simulink model "r_1f_2x_4a_r340"  (Simulink 7.2) (continued)
== system r_1f_2x_4a_r340 == (continued)
      link override r_2x_8a_r320b_1_instance_id_user_data_out: arith_type=Unsigned  n_bits=32  bin_pt=0  period=1
      link override r_2x_8a_r320b_instance_id_user_data_out: arith_type=Unsigned  n_bits=32  bin_pt=0  period=1
      link override r_4f_1x_16a_r322h_instance_id_user_data_out: arith_type=Unsigned  n_bits=32  bin_pt=0  period=1
      link override r_4f_1x_16a_r322i_instance_id_user_data_out: arith_type=Unsigned  n_bits=32  bin_pt=0  period=1
      link override r_4f_1x_16a_r322j_inst_xeng_id0_user_data_out: arith_type=Unsigned  n_bits=32  bin_pt=0  period=1
      link override r_4f_1x_16a_r322k_inst_xeng_id0_user_data_out: arith_type=Unsigned  n_bits=32  bin_pt=0  period=1
      link override r_4f_1x_16a_r322l_inst_xeng_id0_user_data_out: arith_type=Unsigned  n_bits=32  bin_pt=0  period=1
      link override r_4f_1x_16a_r322m_inst_xeng_id0_user_data_out: arith_type=Unsigned  n_bits=32  bin_pt=0  period=1
      link override r_4f_2x_16a_r320b_instance_id_user_data_out: arith_type=Unsigned  n_bits=32  bin_pt=0  period=1
      link override r_4f_2x_16a_r321a_instance_id_user_data_out: arith_type=Unsigned  n_bits=32  bin_pt=0  period=1
      link override r_4f_2x_16a_r322a_instance_id_user_data_out: arith_type=Unsigned  n_bits=32  bin_pt=0  period=1
      link override r_4f_2x_16a_r322b_instance_id_user_data_out: arith_type=Unsigned  n_bits=32  bin_pt=0  period=1
      link override r_4f_2x_16a_r322c_instance_id_user_data_out: arith_type=Unsigned  n_bits=32  bin_pt=0  period=1
      link override r_4f_2x_16a_r322d_instance_id_user_data_out: arith_type=Unsigned  n_bits=32  bin_pt=0  period=1
      link override r_4f_2x_16a_r322e_instance_id_user_data_out: arith_type=Unsigned  n_bits=32  bin_pt=0  period=1
      link override r_4f_2x_16a_r322f_instance_id_user_data_out: arith_type=Unsigned  n_bits=32  bin_pt=0  period=1
      link override r_4f_2x_16a_r322g_instance_id_user_data_out: arith_type=Unsigned  n_bits=32  bin_pt=0  period=1
      link override r_4f_2x_16a_r322h_instance_id_user_data_out: arith_type=Unsigned  n_bits=32  bin_pt=0  period=1
      link override r_4f_2x_16a_r322m_inst_xeng_id1_user_data_out: arith_type=Unsigned  n_bits=32  bin_pt=0  period=1
      link override r_4f_2x_16a_r323a_inst_xeng_id1_user_data_out: arith_type=Unsigned  n_bits=32  bin_pt=0  period=1
      link override r_4f_2x_16a_r330a_inst_xeng_id1_user_data_out: arith_type=Unsigned  n_bits=32  bin_pt=0  period=1
      link override r_4f_2x_16a_r330b_inst_xeng_id1_user_data_out: arith_type=Unsigned  n_bits=32  bin_pt=0  period=1
      link override r_r311b_xaui_test_instance_id_user_data_out: arith_type=Unsigned  n_bits=32  bin_pt=0  period=1
  last_missing_ant0 : xps_library/software register  Tag=xps:sw_reg  SystemSampleTime=-1  io_dir=To Processor  arith_type=Unsigned  bitwidth=32  bin_pt=0  sample_period=1  gw_name=r_1f_2x_4a_r339_last_missing_ant0_user_data_in
      link override convert: arith_type=Unsigned  n_bits=32  bin_pt=0
  last_missing_ant1 : xps_library/software register  Tag=xps:sw_reg  SystemSampleTime=-1  io_dir=To Processor  arith_type=Unsigned  bitwidth=32  bin_pt=0  sample_period=1  gw_name=r_1f_2x_4a_r339_last_missing_ant1_user_data_in
      link override convert: arith_type=Unsigned  n_bits=32  bin_pt=0
  led0_xaui_fault : xps_library/gpio  Tag=xps:gpio  SystemSampleTime=-1  io_group=ROACH:led  io_dir=out  arith_type=Boolean  bitwidth=1  bin_pt=0  bit_index=0  sample_period=1  use_single_ended=off  use_ddr=off  reg_iob=off  reg_clk_phase=0  termination=None
      link override convert: arith_type=Boolean  n_bits=1  bin_pt=0
  led1_xaui_sync : xps_library/gpio  Tag=xps:gpio  SystemSampleTime=-1  io_group=ROACH:led  io_dir=out  arith_type=Boolean  bitwidth=1  bin_pt=0  bit_index=1  sample_period=1  use_single_ended=off  use_ddr=off  reg_iob=off  reg_clk_phase=0  termination=None
      link override convert: arith_type=Boolean  n_bits=1  bin_pt=0
  led2_x_sync : xps_library/gpio  Tag=xps:gpio  SystemSampleTime=-1  io_group=ROACH:led  io_dir=out  arith_type=Boolean  bitwidth=1  bin_pt=0  bit_index=2  sample_period=1  use_single_ended=off  use_ddr=off  reg_iob=off  reg_clk_phase=0  termination=None
      link override convert: arith_type=Boolean  n_bits=1  bin_pt=0
  led3_new_acc : xps_library/gpio  Tag=xps:gpio  SystemSampleTime=-1  io_group=ROACH:led  io_dir=out  arith_type=Boolean  bitwidth=1  bin_pt=0  bit_index=3  sample_period=1  use_single_ended=off  use_ddr=off  reg_iob=off  reg_clk_phase=0  termination=None
      link override convert: arith_type=Boolean  n_bits=1  bin_pt=0
  loop_cnt0 : xps_library/software register  Tag=xps:sw_reg  SystemSampleTime=-1  io_dir=To Processor  arith_type=Unsigned  bitwidth=32  bin_pt=0  sample_period=1  gw_name=r_1f_2x_4a_r339_loop_cnt0_user_data_in
      link override convert: arith_type=Unsigned  n_bits=32  bin_pt=0
  loop_err_cnt0 : xps_library/software register  Tag=xps:sw_reg  SystemSampleTime=-1  io_dir=To Processor  arith_type=Unsigned  bitwidth=32  bin_pt=0  sample_period=1  gw_name=r_1f_2x_4a_r339_loop_err_cnt0_user_data_in
      link override convert: arith_type=Unsigned  n_bits=32  bin_pt=0
  loopback_fifo0 : Subsystem
  loopback_mux0 : Subsystem  mask{timeout_bits=27, payload_len=32, fifo_latency=4}
      mask prompts: timeout_bits "Timeout (clock cycles, 2^?)"; payload_len "Expected payload length (in 64bit words)"; fifo_latency "Input pkt_fifo latencies"
      mask init:
      | payload_bits = ceil(log2(payload_len)+1);
      | fmtstr = sprintf('Payload Len: %i\nFIFO latencies: %i.', payload_len,fifo_latency);
      | set_param(gcb, 'AttributesFormatString', fmtstr);
      settings: AttributesFormatString=|
      | Payload Len: 32
      | FIFO latencies: 4.
  master_reset_sim2 : Constant  Value=0  OutDataType=sfix(16)  OutScaling=2^0
  master_reset_sim4 : Constant  Value=2  OutDataType=sfix(16)  OutScaling=2^0
  master_reset_sim5 : Constant  Value=0  OutDataType=sfix(16)  OutScaling=2^0
  miss_cnt0 : Subsystem  mask{n_ants_bits=2, xeng_int_bits=7}
      mask prompts: n_ants_bits "Number of antennas in system (2^?)"; xeng_int_bits "X engine integration time (2^?)"
  miss_cnt1 : Subsystem  mask{n_ants_bits=2, xeng_int_bits=7}
      mask prompts: n_ants_bits "Number of antennas in system (2^?)"; xeng_int_bits "X engine integration time (2^?)"
  pkt_reord0 : Subsystem  mask{ant_bits=2, xeng_bits=3, chan_bits=9, x_int_bits=7, window_bits=3, min_sync_bits=27}
      mask prompts: ant_bits "Maximum Number of Antennas (2^?):"; xeng_bits "Number of Xengines in the system (2^?)"; chan_bits "Number of frequency channels in the system (2^?)"; x_int_bits "X Engine Integration Length (2^?):"; window_bits "Number of Windows to Buffer (2^?):"; min_sync_bits "Minimum sync period (2^n)"
      mask init:
      | %sync_bits=max([min_sync_bits,chan_bits+x_int_bits+ant_bits-xeng_bits]);
      | sync_bits=chan_bits+x_int_bits+ant_bits-xeng_bits;
      | fmtstr = sprintf('Sync period: 2^%d\nPayload Len: %i', sync_bits,2^(x_int_bits - 2));
      | set_param(gcb, 'AttributesFormatString', fmtstr);
      settings: AttributesFormatString=|
      | Sync period: 2^15
      | Payload Len: 32
  pkt_reord1 : Subsystem  mask{ant_bits=2, xeng_bits=3, chan_bits=9, x_int_bits=7, window_bits=3, min_sync_bits=27}
      mask prompts: ant_bits "Maximum Number of Antennas (2^?):"; xeng_bits "Number of Xengines in the system (2^?)"; chan_bits "Number of frequency channels in the system (2^?)"; x_int_bits "X Engine Integration Length (2^?):"; window_bits "Number of Windows to Buffer (2^?):"; min_sync_bits "Minimum sync period (2^n)"
      mask init: same as r_1f_2x_4a_r340/pkt_reord0
      settings: AttributesFormatString=|
      | Sync period: 2^15
      | Payload Len: 32
  pkt_reord_cnt0 : xps_library/software register  Tag=xps:sw_reg  SystemSampleTime=-1  io_dir=To Processor  arith_type=Unsigned  bitwidth=32  bin_pt=0  sample_period=1  gw_name=r_1f_2x_4a_r339_pkt_reord_cnt0_user_data_in
      link override convert: arith_type=Unsigned  n_bits=32  bin_pt=0
  pkt_reord_cnt1 : xps_library/software register  Tag=xps:sw_reg  SystemSampleTime=-1  io_dir=To Processor  arith_type=Unsigned  bitwidth=32  bin_pt=0  sample_period=1  gw_name=r_1f_2x_4a_r339_pkt_reord_cnt1_user_data_in
      link override convert: arith_type=Unsigned  n_bits=32  bin_pt=0
  pkt_reord_err0 : xps_library/software register  Tag=xps:sw_reg  SystemSampleTime=-1  io_dir=To Processor  arith_type=Unsigned  bitwidth=32  bin_pt=0  sample_period=1  gw_name=r_1f_2x_4a_r339_pkt_reord_err0_user_data_in
      link override convert: arith_type=Unsigned  n_bits=32  bin_pt=0
  pkt_reord_err1 : xps_library/software register  Tag=xps:sw_reg  SystemSampleTime=-1  io_dir=To Processor  arith_type=Unsigned  bitwidth=32  bin_pt=0  sample_period=1  gw_name=r_1f_2x_4a_r339_pkt_reord_err1_user_data_in
      link override convert: arith_type=Unsigned  n_bits=32  bin_pt=0
  posedge2 : casper_library/Misc/posedge  Tag=Rising Edge Detector  SystemSampleTime=-1
  prep_snap_rx0 : Subsystem  mask{chan_bits=9, xeng_bits=3}
      mask prompts: chan_bits "Number of frequency channels in system (2^?)"; xeng_bits "Number of X engines in the system (2^?)"
  pulse_ext : Subsystem  mask{pulse_len=2^23}
      mask prompts: pulse_len "Length of Pulse"
      mask init:
      | bits = ceil(log2(pulse_len));
  pulse_ext1 : Subsystem  mask{pulse_len=2^23}
      mask prompts: pulse_len "Length of Pulse"
      mask init:
      | bits = ceil(log2(pulse_len));
  pulse_ext2 : Subsystem  mask{pulse_len=2^23}
      mask prompts: pulse_len "Length of Pulse"
      mask init:
      | bits = ceil(log2(pulse_len));
  pulse_ext3 : Subsystem  mask{pulse_len=2^23}
      mask prompts: pulse_len "Length of Pulse"
      mask init:
      | bits = ceil(log2(pulse_len));
  rcs : Subsystem  AncestorBlock=casper_library/Misc/revision_control  mask(revision_control){rev=339, error_on_dirty=off, error_on_norcs=off}
      mask prompts: rev "User revision number"; error_on_dirty "Error on out-of-date"; error_on_norcs "Error on no revision control"
      mask init:
      | revision_control_init( gcb, ...
      |   'rev', rev, ...
      |   'error_on_dirty', error_on_dirty, ...
      |   'error_on_norcs', error_on_norcs);
  rx_cnt0 : xps_library/software register  Tag=xps:sw_reg  SystemSampleTime=-1  io_dir=To Processor  arith_type=Unsigned  bitwidth=32  bin_pt=0  sample_period=1  gw_name=r_1f_2x_4a_r339_rx_cnt0_user_data_in
      link override convert: arith_type=Unsigned  n_bits=32  bin_pt=0
  rx_err_cnt0 : xps_library/software register  Tag=xps:sw_reg  SystemSampleTime=-1  io_dir=To Processor  arith_type=Unsigned  bitwidth=32  bin_pt=0  sample_period=1  gw_name=r_1f_2x_4a_r339_rx_err_cnt0_user_data_in
      link override convert: arith_type=Unsigned  n_bits=32  bin_pt=0
  rx_pkt_fifo0 : Subsystem
      settings: AttributesFormatString=|
      | Max 1024 data words.
      | Max 32 pkts.
  snap_descramble0 : Subsystem  mask(snap_circ){nsamples=11}
      mask prompts: nsamples "Number of Samples (2^?)"
      mask help:
      | Backwards compatible with the normal snap block, but with the following upgrades:
      |  1) Ability to offset the trigger. Optionally delay the fabric trigger by a user-specified number of valids.
      |  2) 'Addr' register's MSb now indicates 'busy capturing'.
      |  3) Ability to capture continuously until a stop pulse is received. Data is captured into BRAM continuously, with old data being overwritten until a stop command is received. Then stop capturing when memory is filled. This enables you to get some data before and some data after the stop pulse. Use the 'tr_en_cnt' register to determine where you are in your vector.
  snap_gbe_rx0 : Subsystem  AncestorBlock=casper_library/Scopes/snap64  mask(snap64){nsamples=11}
      mask prompts: nsamples "No. of samples(2^?)"
      mask help:
      | The <i>snap</i> block provides a packaged solution to capturing data from
      | the FPGA fabric and making it accessible from the CPU.  The block comes in
      | 2 flavors: <i>snap</i> (which captures to a 32 bit wide Shared BRAM), and
      | <i>snap64</i> (which captures to 2x32 bit wide Shared BRAMs to effect a
      | 64 bit capture).
      mask Help: eval('xlWeb([getenv(''MLIB_ROOT''), ''\casper_library\doc\snap_usage.html''])')
  snap_gbe_tx0 : Subsystem  AncestorBlock=casper_library/Scopes/snap64  mask(snap64){nsamples=11}
      mask prompts: nsamples "No. of samples(2^?)"
      mask help:
      | The <i>snap</i> block provides a packaged solution to capturing data from
      | the FPGA fabric and making it accessible from the CPU.  The block comes in
      | 2 flavors: <i>snap</i> (which captures to a 32 bit wide Shared BRAM), and
      | <i>snap64</i> (which captures to 2x32 bit wide Shared BRAMs to effect a
      | 64 bit capture).
      mask Help: eval('xlWeb([getenv(''MLIB_ROOT''), ''\casper_library\doc\snap_usage.html''])')
  snap_rx0 : Subsystem  AncestorBlock=casper_library/Scopes/snap64  mask(snap64){nsamples=12}
      mask prompts: nsamples "No. of samples(2^?)"
      mask help:
      | The <i>snap</i> block provides a packaged solution to capturing data from
      | the FPGA fabric and making it accessible from the CPU.  The block comes in
      | 2 flavors: <i>snap</i> (which captures to a 32 bit wide Shared BRAM), and
      | <i>snap64</i> (which captures to 2x32 bit wide Shared BRAMs to effect a
      | 64 bit capture).
      mask Help: eval('xlWeb([getenv(''MLIB_ROOT''), ''\casper_library\doc\snap_usage.html''])')
  snap_vacc0 : Subsystem  AncestorBlock=casper_library/Scopes/snap  mask(snap){nsamples=11}
      mask prompts: nsamples "No. of samples(2^?)"
      mask help:
      | The <i>snap</i> block provides a packaged solution to capturing data from
      | the FPGA fabric and making it accessible from the CPU.  The block comes in
      | 2 flavors: <i>snap</i> (which captures to a 32 bit wide Shared BRAM), and
      | <i>snap64</i> (which captures to 2x32 bit wide Shared BRAMs to effect a
      | 64 bit capture).
      mask Help: eval('xlWeb([getenv(''MLIB_ROOT''), ''\doc\htmlblocks\sys_snap\sys_snap.html''])')
  snap_vacc1 : Subsystem  AncestorBlock=casper_library/Scopes/snap  mask(snap){nsamples=11}
      mask prompts: nsamples "No. of samples(2^?)"
      mask help:
      | The <i>snap</i> block provides a packaged solution to capturing data from
      | the FPGA fabric and making it accessible from the CPU.  The block comes in
      | 2 flavors: <i>snap</i> (which captures to a 32 bit wide Shared BRAM), and
      | <i>snap64</i> (which captures to 2x32 bit wide Shared BRAMs to effect a
      | 64 bit capture).
      mask Help: eval('xlWeb([getenv(''MLIB_ROOT''), ''\doc\htmlblocks\sys_snap\sys_snap.html''])')
  snap_xaui0 : Subsystem  mask{nsamples=13}
      mask prompts: nsamples "Number of Samples (2^?)"
  snap_xeng0 : Subsystem  mask(snap_circ){nsamples=11}
      mask prompts: nsamples "Number of Samples (2^?)"
      mask help:
      | Backwards compatible with the normal snap block, but with the following upgrades:
      |  1) Ability to offset the trigger. Optionally delay the fabric trigger by a user-specified number of valids.
      |  2) 'Addr' register's MSb now indicates 'busy capturing'.
      |  3) Ability to capture continuously until a stop pulse is received. Data is captured into BRAM continuously, with old data being overwritten until a stop command is received. Then stop capturing when memory is filled. This enables you to get some data before and some data after the stop pulse. Use the 'tr_en_cnt' register to determine where you are in your vector.
  tvg_sel : xps_library/software register  Tag=xps:sw_reg  SystemSampleTime=-1  io_dir=From Processor  arith_type=Unsigned  bitwidth=32  bin_pt=0  sample_period=1  gw_name=r_1f_2x_4a_r339_tvg_sel_user_data_out
      link override r_2x_8a_r310b_tvg_sel1_user_data_out: arith_type=Unsigned  n_bits=32  bin_pt=0  period=1
      link override r_2x_8a_r311a_tvg_sel1_user_data_out: arith_type=Unsigned  n_bits=32  bin_pt=0  period=1
      link override r_2x_8a_r311b_1_tvg_sel_user_data_out: arith_type=Unsigned  n_bits=32  bin_pt=0  period=1
      link override r_2x_8a_r311b_tvg_sel_user_data_out: arith_type=Unsigned  n_bits=32  bin_pt=0  period=1
      link override r_2x_8a_r320a_1_tvg_sel_user_data_out: arith_type=Unsigned  n_bits=32  bin_pt=0  period=1
      link override r_2x_8a_r320b_1_tvg_sel_user_data_out: arith_type=Unsigned  n_bits=32  bin_pt=0  period=1
      link override r_2x_8a_r320b_tvg_sel_user_data_out: arith_type=Unsigned  n_bits=32  bin_pt=0  period=1
      link override r_4f_1x_16a_r322h_tvg_sel_user_data_out: arith_type=Unsigned  n_bits=32  bin_pt=0  period=1
      link override r_4f_1x_16a_r322i_tvg_sel_user_data_out: arith_type=Unsigned  n_bits=32  bin_pt=0  period=1
      link override r_4f_1x_16a_r322j_tvg_sel_user_data_out: arith_type=Unsigned  n_bits=32  bin_pt=0  period=1
      link override r_4f_1x_16a_r322k_tvg_sel_user_data_out: arith_type=Unsigned  n_bits=32  bin_pt=0  period=1
      link override r_4f_1x_16a_r322l_tvg_sel_user_data_out: arith_type=Unsigned  n_bits=32  bin_pt=0  period=1
      link override r_4f_1x_16a_r322m_tvg_sel_user_data_out: arith_type=Unsigned  n_bits=32  bin_pt=0  period=1
      link override r_4f_2x_16a_r320b_tvg_sel_user_data_out: arith_type=Unsigned  n_bits=32  bin_pt=0  period=1
      link override r_4f_2x_16a_r321a_tvg_sel_user_data_out: arith_type=Unsigned  n_bits=32  bin_pt=0  period=1
      link override r_4f_2x_16a_r322a_tvg_sel_user_data_out: arith_type=Unsigned  n_bits=32  bin_pt=0  period=1
      link override r_4f_2x_16a_r322b_tvg_sel_user_data_out: arith_type=Unsigned  n_bits=32  bin_pt=0  period=1
      link override r_4f_2x_16a_r322c_tvg_sel_user_data_out: arith_type=Unsigned  n_bits=32  bin_pt=0  period=1
      link override r_4f_2x_16a_r322d_tvg_sel_user_data_out: arith_type=Unsigned  n_bits=32  bin_pt=0  period=1
      link override r_4f_2x_16a_r322e_tvg_sel_user_data_out: arith_type=Unsigned  n_bits=32  bin_pt=0  period=1
      link override r_4f_2x_16a_r322f_tvg_sel_user_data_out: arith_type=Unsigned  n_bits=32  bin_pt=0  period=1
      link override r_4f_2x_16a_r322g_tvg_sel_user_data_out: arith_type=Unsigned  n_bits=32  bin_pt=0  period=1
      link override r_4f_2x_16a_r322h_tvg_sel_user_data_out: arith_type=Unsigned  n_bits=32  bin_pt=0  period=1
      link override r_4f_2x_16a_r322m_tvg_sel_user_data_out: arith_type=Unsigned  n_bits=32  bin_pt=0  period=1
      link override r_4f_2x_16a_r323a_tvg_sel_user_data_out: arith_type=Unsigned  n_bits=32  bin_pt=0  period=1
      link override r_4f_2x_16a_r330a_tvg_sel_user_data_out: arith_type=Unsigned  n_bits=32  bin_pt=0  period=1
      link override r_4f_2x_16a_r330b_tvg_sel_user_data_out: arith_type=Unsigned  n_bits=32  bin_pt=0  period=1
      link override r_r311b_xaui_test_tvg_sel_user_data_out: arith_type=Unsigned  n_bits=32  bin_pt=0  period=1
      link override test_x_eng_nw_sim_pkt_4a_10_1_tvg_sel_user_data_out: arith_type=Unsigned  n_bits=32  bin_pt=0  period=1
      link override test_x_eng_sim_packet_4a_10_1_tvg_sel_user_data_out: arith_type=Unsigned  n_bits=32  bin_pt=0  period=1
      link override test_x_eng_sim_pkt_4a_10_1_new_tvg_sel_user_data_out: arith_type=Unsigned  n_bits=32  bin_pt=0  period=1
      link override xflow_sim_8a_pt2_tvg_sel_user_data_out: arith_type=Unsigned  n_bits=32  bin_pt=0  period=1
      link override xflow_sim_8a_tvg_sel_user_data_out: arith_type=Unsigned  n_bits=32  bin_pt=0  period=1
      link override xflow_sim_tvg_sel_user_data_out: arith_type=Unsigned  n_bits=32  bin_pt=0  period=1
  vacc0 : Subsystem  mask{ants_bits=2, freq_bits=9, xeng_bits=3, which_qdr=qdr0}
      mask prompts: ants_bits "Number of antennas (2^n)"; freq_bits "Number of frequency channels (2^n)"; xeng_bits "Number of X engines (2^n)"; which_qdr "QDR"
      mask help: Hardcoded for X engines with a demux of 8!
      mask init:
      | vector_len = (2^ants_bits)*(2^ants_bits +1) /2 *8 * 2^(freq_bits-xeng_bits)
      | set_param([gcb, '/qv/qdr'], 'which_qdr', which_qdr);
      | fmtstr = sprintf('Vector Length: %d', vector_len);
      | set_param(gcb, 'AttributesFormatString', fmtstr);
      settings: AttributesFormatString=Vector Length: 5120
      mask StyleString: edit,edit,edit,popup(qdr0|qdr1)
  vacc1 : Subsystem  mask{ants_bits=2, freq_bits=9, xeng_bits=3, which_qdr=qdr1}
      mask prompts: ants_bits "Number of antennas (2^n)"; freq_bits "Number of frequency channels (2^n)"; xeng_bits "Number of X engines (2^n)"; which_qdr "QDR"
      mask help: Hardcoded for X engines with a demux of 8!
      mask init: same as r_1f_2x_4a_r340/vacc0
      settings: AttributesFormatString=Vector Length: 5120
      mask StyleString: edit,edit,edit,popup(qdr0|qdr1)
  vacc_cnt0 : xps_library/software register  Tag=xps:sw_reg  SystemSampleTime=-1  io_dir=To Processor  arith_type=Unsigned  bitwidth=32  bin_pt=0  sample_period=1  gw_name=r_1f_2x_4a_r339_vacc_cnt0_user_data_in
      link override convert: arith_type=Unsigned  n_bits=32  bin_pt=0
  vacc_cnt1 : xps_library/software register  Tag=xps:sw_reg  SystemSampleTime=-1  io_dir=To Processor  arith_type=Unsigned  bitwidth=32  bin_pt=0  sample_period=1  gw_name=r_1f_2x_4a_r339_vacc_cnt1_user_data_in
      link override convert: arith_type=Unsigned  n_bits=32  bin_pt=0
  vacc_err_cnt0 : xps_library/software register  Tag=xps:sw_reg  SystemSampleTime=-1  io_dir=To Processor  arith_type=Unsigned  bitwidth=32  bin_pt=0  sample_period=1  gw_name=r_1f_2x_4a_r339_vacc_err_cnt0_user_data_in
      link override convert: arith_type=Unsigned  n_bits=32  bin_pt=0
  vacc_err_cnt1 : xps_library/software register  Tag=xps:sw_reg  SystemSampleTime=-1  io_dir=To Processor  arith_type=Unsigned  bitwidth=32  bin_pt=0  sample_period=1  gw_name=r_1f_2x_4a_r339_vacc_err_cnt1_user_data_in
      link override convert: arith_type=Unsigned  n_bits=32  bin_pt=0
  vacc_ld_status0 : xps_library/software register  Tag=xps:sw_reg  SystemSampleTime=-1  io_dir=To Processor  arith_type=Unsigned  bitwidth=32  bin_pt=0  sample_period=1  gw_name=r_1f_2x_4a_r339_vacc_ld_status0_user_data_in
      link override convert: arith_type=Unsigned  n_bits=32  bin_pt=0
  vacc_ld_status1 : xps_library/software register  Tag=xps:sw_reg  SystemSampleTime=-1  io_dir=To Processor  arith_type=Unsigned  bitwidth=32  bin_pt=0  sample_period=1  gw_name=r_1f_2x_4a_r339_vacc_ld_status1_user_data_in
      link override convert: arith_type=Unsigned  n_bits=32  bin_pt=0
  vacc_mcnt_h0 : xps_library/software register  Tag=xps:sw_reg  SystemSampleTime=-1  io_dir=To Processor  arith_type=Unsigned  bitwidth=32  bin_pt=0  sample_period=1  gw_name=r_1f_2x_4a_r339_vacc_mcnt_h0_user_data_in
      link override convert: arith_type=Unsigned  n_bits=32  bin_pt=0
  vacc_mcnt_h1 : xps_library/software register  Tag=xps:sw_reg  SystemSampleTime=-1  io_dir=To Processor  arith_type=Unsigned  bitwidth=32  bin_pt=0  sample_period=1  gw_name=r_1f_2x_4a_r339_vacc_mcnt_h1_user_data_in
      link override convert: arith_type=Unsigned  n_bits=32  bin_pt=0
  vacc_mcnt_l0 : xps_library/software register  Tag=xps:sw_reg  SystemSampleTime=-1  io_dir=To Processor  arith_type=Unsigned  bitwidth=32  bin_pt=0  sample_period=1  gw_name=r_1f_2x_4a_r339_vacc_mcnt_l0_user_data_in
      link override convert: arith_type=Unsigned  n_bits=32  bin_pt=0
  vacc_mcnt_l1 : xps_library/software register  Tag=xps:sw_reg  SystemSampleTime=-1  io_dir=To Processor  arith_type=Unsigned  bitwidth=32  bin_pt=0  sample_period=1  gw_name=r_1f_2x_4a_r339_vacc_mcnt_l1_user_data_in
      link override convert: arith_type=Unsigned  n_bits=32  bin_pt=0
  vacc_monitor : Subsystem
  vacc_monitor1 : Subsystem
  vacc_time_lsw : xps_library/software register  Tag=xps:sw_reg  SystemSampleTime=-1  io_dir=From Processor  arith_type=Unsigned  bitwidth=32  bin_pt=0  sample_period=1  gw_name=r_1f_2x_4a_r339_vacc_time_lsw_user_data_out
      link override poco_cd_tr0_ld_time_lsb_user_data_out: arith_type=Unsigned  n_bits=32  bin_pt=0  period=1
      link override poco_dram_vacc_r001_cd_tr0_ld_time_lsb_user_data_out: arith_type=Unsigned  n_bits=32  bin_pt=0  period=1
      link override poco_dram_vacc_r002_cd_tr0_ld_time_lsb_user_data_out: arith_type=Unsigned  n_bits=32  bin_pt=0  period=1
      link override poco_dram_vacc_r003_cd_tr0_ld_time_lsb_user_data_out: arith_type=Unsigned  n_bits=32  bin_pt=0  period=1
      link override poco_dram_vacc_r004_cd_tr0_ld_time_lsb_user_data_out: arith_type=Unsigned  n_bits=32  bin_pt=0  period=1
      link override poco_dram_vacc_r005_cd_tr0_ld_time_lsb_user_data_out: arith_type=Unsigned  n_bits=32  bin_pt=0  period=1
      link override poco_dram_vacc_r006_cd_tr0_ld_time_lsb_user_data_out: arith_type=Unsigned  n_bits=32  bin_pt=0  period=1
      link override poco_r006_cd_tr0_ld_time_lsb_user_data_out: arith_type=Unsigned  n_bits=32  bin_pt=0  period=1
      link override poco_red_cd_ld_time_lsb_user_data_out: arith_type=Unsigned  n_bits=32  bin_pt=0  period=1
      link override poco_ref_roach_adc0_cd_ld_time_lsb_user_data_out: arith_type=Unsigned  n_bits=32  bin_pt=0  period=1
      link override poco_ref_roach_reduced_adc0_cd_ld_time_lsb_user_data_out: arith_type=Unsigned  n_bits=32  bin_pt=0  period=1
      link override poco_roach_cd_tr0_ld_time_lsb_user_data_out: arith_type=Unsigned  n_bits=32  bin_pt=0  period=1
      link override poco_roach_r006_cd_tr0_ld_time_lsb_user_data_out: arith_type=Unsigned  n_bits=32  bin_pt=0  period=1
      link override poco_roach_r007_cd_tr0_ld_time_lsb_user_data_out: arith_type=Unsigned  n_bits=32  bin_pt=0  period=1
      link override poco_roach_red_adc0_cd_ld_time_lsb_user_data_out: arith_type=Unsigned  n_bits=32  bin_pt=0  period=1
      link override poco_roach_vacc_r006_cd_tr0_ld_time_lsb_user_data_out: arith_type=Unsigned  n_bits=32  bin_pt=0  period=1
  vacc_time_msw : xps_library/software register  Tag=xps:sw_reg  SystemSampleTime=-1  io_dir=From Processor  arith_type=Unsigned  bitwidth=32  bin_pt=0  sample_period=1  gw_name=r_1f_2x_4a_r339_vacc_time_msw_user_data_out
      link override poco_cd_tr0_ld_time_msb_user_data_out: arith_type=Unsigned  n_bits=32  bin_pt=0  period=1
      link override poco_dram_vacc_r001_cd_tr0_ld_time_msb_user_data_out: arith_type=Unsigned  n_bits=32  bin_pt=0  period=1
      link override poco_dram_vacc_r002_cd_tr0_ld_time_msb_user_data_out: arith_type=Unsigned  n_bits=32  bin_pt=0  period=1
      link override poco_dram_vacc_r003_cd_tr0_ld_time_msb_user_data_out: arith_type=Unsigned  n_bits=32  bin_pt=0  period=1
      link override poco_dram_vacc_r004_cd_tr0_ld_time_msb_user_data_out: arith_type=Unsigned  n_bits=32  bin_pt=0  period=1
      link override poco_dram_vacc_r005_cd_tr0_ld_time_msb_user_data_out: arith_type=Unsigned  n_bits=32  bin_pt=0  period=1
      link override poco_dram_vacc_r006_cd_tr0_ld_time_msb_user_data_out: arith_type=Unsigned  n_bits=32  bin_pt=0  period=1
      link override poco_r006_cd_tr0_ld_time_msb_user_data_out: arith_type=Unsigned  n_bits=32  bin_pt=0  period=1
      link override poco_red_cd_ld_time_msb_user_data_out: arith_type=Unsigned  n_bits=32  bin_pt=0  period=1
      link override poco_ref_roach_adc0_cd_ld_time_msb_user_data_out: arith_type=Unsigned  n_bits=32  bin_pt=0  period=1
      link override poco_ref_roach_reduced_adc0_cd_ld_time_msb_user_data_out: arith_type=Unsigned  n_bits=32  bin_pt=0  period=1
      link override poco_roach_cd_tr0_ld_time_msb_user_data_out: arith_type=Unsigned  n_bits=32  bin_pt=0  period=1
      link override poco_roach_r006_cd_tr0_ld_time_msb_user_data_out: arith_type=Unsigned  n_bits=32  bin_pt=0  period=1
      link override poco_roach_r007_cd_tr0_ld_time_msb_user_data_out: arith_type=Unsigned  n_bits=32  bin_pt=0  period=1
      link override poco_roach_red_adc0_cd_ld_time_msb_user_data_out: arith_type=Unsigned  n_bits=32  bin_pt=0  period=1
      link override poco_roach_vacc_r006_cd_tr0_ld_time_msb_user_data_out: arith_type=Unsigned  n_bits=32  bin_pt=0  period=1
  vacc_tvg0 : Subsystem  mask{len=5120}
      mask prompts: len "Number of Vectors"
      mask init:
      | fmtstr = sprintf('Vector Length: %d', len);
      | set_param(gcb, 'AttributesFormatString', fmtstr);
      settings: AttributesFormatString=Vector Length: 5120
  vacc_tvg1 : Subsystem  mask{len=5120}
      mask prompts: len "Number of Vectors"
      mask init:
      | fmtstr = sprintf('Vector Length: %d', len);
      | set_param(gcb, 'AttributesFormatString', fmtstr);
      settings: AttributesFormatString=Vector Length: 5120
  xaui_cnt0 : xps_library/software register  Tag=xps:sw_reg  SystemSampleTime=-1  io_dir=To Processor  arith_type=Unsigned  bitwidth=32  bin_pt=0  sample_period=1  gw_name=r_1f_2x_4a_r339_xaui_cnt0_user_data_in
      link override convert: arith_type=Unsigned  n_bits=32  bin_pt=0
  xaui_err0 : xps_library/software register  Tag=xps:sw_reg  SystemSampleTime=-1  io_dir=To Processor  arith_type=Unsigned  bitwidth=32  bin_pt=0  sample_period=1  gw_name=r_1f_2x_4a_r339_xaui_err0_user_data_in
      link override convert: arith_type=Unsigned  n_bits=32  bin_pt=0
  xaui_pack0 : Subsystem  mask{payload_len=32, xeng_bits=3, skip_xeng_bits=1, skip_ant_bits=0}
      mask prompts: payload_len "Payload Length (in 64 bit words)"; xeng_bits "Number of X engines (2^?)"; skip_xeng_bits "X engines per IP address (2^?)"; skip_ant_bits "Antennas per XAUI link (2^?)"
      mask help: Packetizes data coming in over a XAUI interface. A packet consists of a 64 bit header (48 bits of "mcnt" and 16 bits of antenna), followed by 64 x "payload" bits of payload. "Mcnt" (master count) is a counter which keeps track of how many packets have been transmitted since the last "mrst".
      mask init:
      | packet_len = payload_len + 1;
      | packet_bits = ceil(log2(packet_len));
      | payload_bits = ceil(log2(payload_len));
  xaui_sync_mcnt0 : xps_library/software register  Tag=xps:sw_reg  SystemSampleTime=-1  io_dir=To Processor  arith_type=Unsigned  bitwidth=32  bin_pt=0  sample_period=1  gw_name=r_1f_2x_4a_r339_xaui_sync_mcnt0_user_data_in
      link override convert: arith_type=Unsigned  n_bits=32  bin_pt=0
  xeng0 : Subsystem  AncestorBlock=casper_library/Correlator/xeng  mask(xeng){n_ants=4, n_bits=4, acc_len=128, demux_factor=8, add_latency=1, mult_latency=2, bram_latency=2, use_ded_mult=1, use_bram_delay=1}
      mask prompts: n_ants "Number of antennas"; n_bits "Bit-width of samples in"; acc_len "Accumulation length"; add_latency "Adder latency"; mult_latency "Multiplier latency"; bram_latency "BRAM latency"; use_ded_mult "Implementation: Multiplier type (0=slices 1=embedded 2=bram)"; use_bram_delay "Implementation: Delay type (0=SLR, 1=BRAM)"
      mask help: CASPER X engine with added internal valid data masking functionality, descramble and integral demux. Based on Aaron Parsons' and Lynn Urry's original X engine design.
      mask init:
      | xeng_init(gcb, ...
      |     'n_ants', n_ants, ...
      |     'n_bits', n_bits, ...
      |     'acc_len', acc_len, ...
      |     'demux_factor', demux_factor, ...
      |     'add_latency', add_latency, ...
      |     'mult_latency', mult_latency, ...
      |     'bram_latency', bram_latency, ...
      |     'use_ded_mult', use_ded_mult, ...
      |     'use_bram_delay', use_bram_delay);
      settings: AttributesFormatString=n_ant=4, bits=4, mult=1, bram=1
      mask StyleString: edit,edit,edit,popup(1|2|4|8),edit,edit,edit,edit,edit
  xeng1 : Subsystem  AncestorBlock=casper_library/Correlator/xeng  mask(xeng){n_ants=4, n_bits=4, acc_len=128, demux_factor=8, add_latency=1, mult_latency=2, bram_latency=2, use_ded_mult=1, use_bram_delay=1}
      mask prompts: n_ants "Number of antennas"; n_bits "Bit-width of samples in"; acc_len "Accumulation length"; add_latency "Adder latency"; mult_latency "Multiplier latency"; bram_latency "BRAM latency"; use_ded_mult "Implementation: Multiplier type (0=slices 1=embedded 2=bram)"; use_bram_delay "Implementation: Delay type (0=SLR, 1=BRAM)"
      mask help: CASPER X engine with added internal valid data masking functionality, descramble and integral demux. Based on Aaron Parsons' and Lynn Urry's original X engine design.
      mask init: same as r_1f_2x_4a_r340/xeng0
      settings: AttributesFormatString=n_ant=4, bits=4, mult=1, bram=1
      mask StyleString: edit,edit,edit,popup(1|2|4|8),edit,edit,edit,edit,edit
  xeng_tvg0 : Subsystem  AncestorBlock=casper_library/Correlator/xeng_tvg  mask{ant_bits=2, x_int_bits=7, sync_period=16}
      mask prompts: ant_bits "Number of Antennas (2^?):"; x_int_bits "X Integration Length (2^?):"; sync_period "Sync Pulse Period (2^?):"
  xeng_tvg1 : Subsystem  AncestorBlock=casper_library/Correlator/xeng_tvg  mask{ant_bits=2, x_int_bits=7, sync_period=16}
      mask prompts: ant_bits "Number of Antennas (2^?):"; x_int_bits "X Integration Length (2^?):"; sync_period "Sync Pulse Period (2^?):"
  wires:
  loopback_mux0/9(loop_re) -> Delay147/1(d), Delay47/1
  loopback_mux0/10(gbe_re) -> Delay148/1(d), Delay48/1
  gbe_err/1(tx_cnt) -> Delay63/1(d)
  Subsystem/1(rx_cnt) -> Delay65/1(d)
  [cnt_rst] -> gbe_err/1(rst)
  [cnt_rst] -> Subsystem/1(rst)
  Subsystem5/1(rx_cnt) -> Delay66/1(d)
  [cnt_rst] -> Subsystem5/1(rst)
  loopback_mux0/2(valid) -> Delay37/1(d), Delay141/1(d), Delay80/1(d)
  loopback_mux0/4(mcnt_out) -> Delay39/1(d), Delay143/1(d)
  loopback_mux0/1(dout) -> Delay36/1(d), Delay140/1(d)
  loopback_mux0/3(eof) -> Delay38/1(d), Delay142/1(d), Delay81/1(d)
  loopback_mux0/5(ant_out) -> Delay35/1(d), Delay144/1(d)
  miss_cnt0/2(err_cnt) -> Delay62/1(d)
  [cnt_rst] -> miss_cnt0/1(rst)
  loopback_fifo0/1(dout) -> loopback_mux0/7(loop_din)
  loopback_fifo0/2(valid) -> Subsystem12/2(we), loopback_mux0/8(loop_valid)
  loopback_fifo0/4(eof_out) -> Subsystem12/3(eof), loopback_mux0/10(loop_eof)
  prep_snap_rx0/1(data_out) -> snap_rx0/1(data_in)
  prep_snap_rx0/2(oob_out) -> snap_rx0/2(oob_in)
  prep_snap_rx0/3(trig_out) -> snap_rx0/3(trig)
  prep_snap_rx0/4(we_out) -> snap_rx0/4(we)
  loopback_fifo0/3(ip_addr) -> loopback_mux0/9(loop_ip)
  loopback_mux0/8(ip) -> Delay146/1(d)
  Delay36/1(q) -> pkt_reord0/1(din), pkt_reord1/1(din)
  Delay37/1(q) -> demux_gbe0/1(we), demux_gbe1/1(we)
  Delay38/1(q) -> pkt_reord0/3(eof), pkt_reord1/3(eof)
  Delay39/1(q) -> pkt_reord0/4(mcnt), demux_gbe0/2(mcnt), demux_gbe1/2(mcnt), pkt_reord1/4(mcnt)
  Delay35/1(q) -> pkt_reord0/5(ant), pkt_reord1/5(ant)
  Subsystem14/1(data) -> Delay41/1(d)
  Delay42/1(q) -> snap_descramble0/2(we)
  Delay43/1(q) -> snap_descramble0/3(trig)
  Delay41/1(q) -> snap_descramble0/1(data_in)
  Subsystem14/2(we) -> Delay42/1(d)
  Subsystem14/3(trig) -> Delay43/1(d)
  Delay40/1(q) -> pkt_reord0/6(flag), pkt_reord1/6(flag)
  gbe_err/2(err_cnt) -> Delay64/1(d)
  Subsystem5/2(err_cnt) -> Delay67/1(d)
  miss_cnt0/1(spectr_cnt) -> Delay61/1(d)
  gbe_tx_cnt0/1 -> Terminator13/1
  gbe_tx_err_cnt0/1 -> Terminator14/1
  gbe_rx_cnt0/1 -> Terminator15/1
  rx_cnt0/1 -> Terminator16/1
  rx_err_cnt0/1 -> Terminator17/1
  pkt_reord_cnt0/1 -> Terminator25/1
  pkt_reord_err0/1 -> Terminator26/1
  Delay61/1(q) -> pkt_reord_cnt0/1
  Delay62/1(q) -> pkt_reord_err0/1
  Delay66/1(q) -> rx_cnt0/1
  Delay67/1(q) -> rx_err_cnt0/1
  Delay63/1(q) -> gbe_tx_cnt0/1
  Delay64/1(q) -> gbe_tx_err_cnt0/1
  Delay65/1(q) -> gbe_rx_cnt0/1
  pkt_reord0/6(mcnt_out) -> Subsystem14/6(max_mcnt), Delay26/1(d)
  pkt_reord0/1(sync_out) -> Delay20/1(d), [x_sync0], Subsystem14/5(sync), miss_cnt0/4(sync)
  pkt_reord0/2(data_out) -> Subsystem14/1(dout), Delay21/1(d)
  pkt_reord0/3(valid_out) -> Subsystem14/2(valid), miss_cnt0/2(valid), Delay22/1(d)
  pkt_reord0/5(rcvd) -> Subsystem14/4(rcvd), miss_cnt0/3(rcvd)
  pkt_reord0/4(flag_out) -> Subsystem14/3(flag)
  err_clear_sim4/1 -> gbe_port/1
  gbe_port/1 -> Delay71/1(d)
  err_clear_sim3/1 -> gbe_ip0/1
  gbe_ip0/1 -> Delay72/1(d)
  XAUI0/1 -> Delay54/1(d)
  XAUI0/4 -> Delay57/1(d)
  XAUI0/5 -> Delay77/1(d)
  xaui_pack0/6(sync) -> Delay31/1(d)
  XAUI0/2 -> Delay55/1(d)
  xaui_pack0/7(mrst) -> Delay34/1(d)
  xaui_pack0/5(discard) -> Delay33/1(d)
  [gbe_base_ip] -> xaui_pack0/5(base_ip)
  xaui_pack0/1(dout) -> Delay9/1(d)
  xaui_pack0/2(valid_out) -> Delay23/1(d)
  xaui_pack0/3(dest_ip) -> Delay10/1(d)
  xaui_pack0/4(eof) -> Delay11/1(d)
  Constant10/1 -> XAUI0/1
  Constant9/1 -> XAUI0/2
  Constant1/1 -> XAUI0/4
  Subsystem9/1(data_out) -> snap_xaui0/1(data_in)
  Subsystem11/1(tx_cnt) -> Delay68/1(d)
  [cnt_rst] -> Subsystem11/1(rst)
  Delay1/1(q) -> xaui_pack0/1(din)
  Delay3/1(q) -> xaui_pack0/2(oob)
  Delay4/1(q) -> xaui_pack0/3(valid_in)
  Delay5/1(q) -> xaui_pack0/4(linkdown_in)
  Delay31/1(q) -> [xaui_sync]
  Delay33/1(q) -> [xaui_pkt_err]
  Delay34/1(q) -> [xaui_mrst]
  XAUI0/3 -> Terminator4/1
  XAUI0/6 -> Terminator5/1
  XAUI0/7 -> Terminator6/1
  Subsystem11/2(err_cnt) -> Delay69/1(d)
  [xaui_pkt_err] -> Subsystem11/5(pkt_err)
  xaui_cnt0/1 -> Terminator21/1
  xaui_err0/1 -> Terminator22/1
  Delay68/1(q) -> xaui_cnt0/1
  Delay69/1(q) -> xaui_err0/1
  Delay71/1(q) -> [gbe_txport]
  Delay72/1(q) -> [gbe_base_ip]
  master_reset_sim4/1 -> acc_len/1
  acc_len/1 -> [acc_len]
  tvg_sel/1 -> Delay89/1(d)
  Delay89/1(q) -> dev_sel2/1, Slice3/1, dev_sel5/1, Slice5/1
  dev_sel2/1 -> [xaui_tvg_sel]
  Slice3/1 -> [descr_tvg_sel]
  dev_sel5/1 -> [xeng_tvg_sel]
  Slice5/1 -> [vacc_tvg_sel]
  err_clear_sim2/1 -> Subsystem2/5(data_sel)
  err_clear_sim6/1 -> Subsystem2/4(valid_sel)
  err_clear_sim7/1 -> Subsystem2/3(inj_vector)
  err_clear_sim8/1 -> Subsystem2/2(inj_counter)
  Pulse Generator1/1 -> Subsystem2/1(new_acc)
  err_clear_sim9/1 -> Subsystem2/6(run)
  Subsystem2/1(Out1) -> tvg_sel/1
  Pulse Generator2/1 -> ctrl/1
  ctrl/1 -> Delay19/1(d)
  dev_sel3/1 -> Delay88/1(d)
  Slice9/1 -> Delay78/1(d)
  Slice7/1 -> posedge2/1
  posedge2/1 -> Delay58/1(d)
  Delay19/1(q) -> dev_sel3/1, Slice7/1, Slice1/1, Slice2/1, Slice8/1, Slice9/1, Slice14/1
  Delay58/1(q) -> [cnt_rst]
  Delay78/1(q) -> [gbe_rst]
  Delay88/1(q) -> [vacc_rst]
  master_reset_sim2/1 -> inst_xeng_id0/1
  inst_xeng_id0/1 -> Delay127/1(d)
  Delay127/1(q) -> dev_sel1/1, dev_sel4/1
  dev_sel1/1 -> [instrument_instance0]
  dev_sel4/1 -> [xeng_id0]
  [gbe_disable] -> Inverter1/1
  Slice1/1 -> Delay60/1(d)
  Delay60/1(q) -> [gbe_disable]
  Subsystem4/1(data_out) -> snap_gbe_rx0/1(data_in)
  Subsystem4/2(oob_out) -> snap_gbe_rx0/2(oob_in)
  Subsystem4/3(trig_out) -> snap_gbe_rx0/3(trig)
  Subsystem4/4(we_out) -> snap_gbe_rx0/4(we)
  rx_pkt_fifo0/1(data) -> loopback_mux0/2(gbe_din)
  rx_pkt_fifo0/2(valid) -> loopback_mux0/3(gbe_valid)
  rx_pkt_fifo0/3(ip_addr) -> loopback_mux0/4(gbe_ip)
  rx_pkt_fifo0/4(eof) -> loopback_mux0/5(gbe_eof)
  xaui_sync_mcnt0/1 -> Terminator3/1
  Delay93/1(q) -> xaui_sync_mcnt0/1
  Subsystem11/3(mcnt_at_sync) -> Delay93/1(d)
  Inverter1/1 -> Logical1/2
  pulse_ext1/1(out) -> led2_x_sync/1
  Delay17/1(q) -> pulse_ext1/1(in)
  [x_sync0] -> Delay17/1(d)
  led2_x_sync/1 -> Terminator30/1
  pulse_ext/1(out) -> led1_xaui_sync/1
  Delay13/1(q) -> pulse_ext/1(in)
  [xaui_sync] -> Delay13/1(d)
  [xaui_link_dn] -> Delay32/1(d)
  pulse_ext3/1(out) -> led0_xaui_fault/1
  led1_xaui_sync/1 -> Terminator18/1
  led0_xaui_fault/1 -> Terminator19/1
  loop_err_cnt0/1 -> Terminator24/1
  [cnt_rst] -> Subsystem12/1(rst)
  Subsystem12/2(err_cnt) -> loop_err_cnt0/1
  loop_cnt0/1 -> Terminator27/1
  Subsystem12/1(rx_cnt) -> loop_cnt0/1
  loopback_fifo0/5(data_rdy) -> loopback_mux0/11(loop_data_rdy)
  Delay140/1(q) -> prep_snap_rx0/1(data_in)
  Delay141/1(q) -> prep_snap_rx0/2(valid_in)
  Delay142/1(q) -> prep_snap_rx0/3(eof_in)
  Delay143/1(q) -> prep_snap_rx0/4(mcnt)
  Delay144/1(q) -> prep_snap_rx0/5(ant)
  Delay145/1(q) -> prep_snap_rx0/6(flag)
  Delay146/1(q) -> prep_snap_rx0/7(ip_in)
  Delay147/1(q) -> prep_snap_rx0/8(loop_ack)
  Delay148/1(q) -> prep_snap_rx0/9(gbe_ack)
  Delay8/1(q) -> Subsystem4/7(bad_frame)
  Delay91/1(q) -> snap_descramble0/4(stop)
  Subsystem14/4(stop) -> Delay91/1(d)
  Constant3/1 -> XAUI0/3
  Constant7/1 -> XAUI0/5
  xeng_tvg0/1(sync_out) -> Delay56/1(d)
  xeng_tvg0/2(data_out) -> Delay73/1(d)
  xeng_tvg0/3(valid_out) -> Delay74/1(d)
  xeng0/1(sync_out) -> snap_xeng0/3(trig), vacc_tvg0/2(sync_in)
  xeng0/2(acc) -> 16b_32b/1(In1)
  xeng0/3(valid) -> snap_xeng0/2(we), vacc_tvg0/4(valid_in)
  [xeng_tvg_sel] -> xeng_tvg0/1(tvg_sel)
  [vacc_tvg_sel] -> vacc_tvg0/1(tvg_sel)
  Delay151/1(q) -> Delay83/1(d)
  Delay20/1(q) -> xeng_tvg0/2(sync)
  Delay21/1(q) -> xeng_tvg0/3(data_in)
  Delay22/1(q) -> xeng_tvg0/4(valid_in)
  Delay26/1(q) -> Delay151/1(d)
  [loopback_mux_rst] -> loopback_mux0/1(rst)
  rx_pkt_fifo0/5(data_rdy) -> loopback_mux0/6(gbe_data_rdy)
  loopback_fifo0/6(overflow) -> Subsystem12/4(overflow)
  gbe_rx_err_cnt0/1 -> Terminator7/1
  Delay2/1(q) -> gbe_rx_err_cnt0/1
  Subsystem/2(rx_err_cnt) -> Delay2/1(d)
  Slice2/1 -> Delay117/1(d)
  Delay117/1(q) -> [loopback_mux_rst]
  pulse_ext2/1(out) -> led3_new_acc/1
  Delay118/1(q) -> pulse_ext2/1(in)
  [new_acc0] -> Delay118/1(d)
  led3_new_acc/1 -> Terminator9/1
  Slice8/1 -> Delay122/1(d)
  Delay122/1(q) -> [gbe_out_rst]
  Constant4/1 -> snap_xeng0/4(stop)
  16b_32b/1(Out1) -> vacc_tvg0/3(data_in), snap_xeng0/1(data_in)
  last_missing_ant0/1 -> Terminator11/1
  Delay130/1(q) -> last_missing_ant0/1
  miss_cnt0/3(last_missed_ant) -> Delay130/1(d)
  Slice14/1 -> Delay100/1(d)
  Delay100/1(q) -> [gbe_out_enable]
  loopback_mux0/6(flag) -> Delay40/1(d), Delay145/1(d)
  loopback_mux0/7(err) -> Delay82/1(d)
  Delay12/1(q) -> Subsystem4/8(overrun)
  [gbe_up] -> Delay87/1(d)
  [gbe_rx] -> Delay86/1(d)
  [gbe_rxip] -> Delay75/1(d)
  [gbe_rxvld] -> Delay76/1(d)
  [gbe_rxdata] -> Delay79/1(d)
  [gbe_rxeof] -> Delay24/1(d)
  Delay87/1(q) -> Subsystem4/1(led_up)
  Delay86/1(q) -> Subsystem4/2(led_rx)
  Delay79/1(q) -> rx_pkt_fifo0/1(d_in)
  Delay76/1(q) -> rx_pkt_fifo0/2(valid_in)
  Delay75/1(q) -> rx_pkt_fifo0/3(dest_ip_0)
  Delay24/1(q) -> rx_pkt_fifo0/4(eof_in)
  vacc_tvg0/1(sync_out) -> vacc0/5(sync_in)
  vacc_tvg0/2(data_out) -> vacc0/6(data_in)
  vacc_tvg0/3(valid_out) -> vacc0/7(we)
  [acc_len] -> vacc0/4(acc_len)
  vacc0/2(data_out) -> snap_vacc0/1(din)
  vacc0/4(valid_out) -> snap_vacc0/2(we)
  xeng0/4(mcnt_out) -> delay5/1
  vacc_cnt0/1 -> Terminator10/1
  vacc_monitor/2(spec_cnt) -> vacc_cnt0/1
  vacc_err_cnt0/1 -> Terminator1/1
  vacc_monitor/1(error_cnt) -> vacc_err_cnt0/1
  vacc0/1(new_acc) -> [new_acc0]
  [cnt_rst] -> vacc_monitor/1(cnt_rst)
  vacc_mcnt_h0/1 -> Terminator2/1
  [vacc_mcnt0] -> Slice/1
  Slice/1 -> vacc_mcnt_h0/1
  vacc_mcnt_l0/1 -> Terminator33/1
  [vacc_mcnt0] -> Slice10/1
  Slice10/1 -> vacc_mcnt_l0/1
  Subsystem9/2(oob_out) -> snap_xaui0/2(oob_in)
  Subsystem9/3(we_out) -> snap_xaui0/3(we)
  Subsystem9/4(trig_out) -> snap_xaui0/4(trig)
  Subsystem9/5(stop) -> snap_xaui0/5(stop)
  snap_vacc0/1(fetch) -> vacc0/9(re)
  vacc0/8(vacc_mcnt) -> [vacc_mcnt0]
  master_reset_sim5/1 -> inst_xeng_id1/1
  inst_xeng_id1/1 -> Delay27/1(d)
  Delay27/1(q) -> dev_sel7/1, dev_sel6/1
  dev_sel6/1 -> [instrument_instance1]
  dev_sel7/1 -> [xeng_id1]
  miss_cnt1/2(err_cnt) -> Delay90/1(d)
  [cnt_rst] -> miss_cnt1/1(rst)
  miss_cnt1/1(spectr_cnt) -> Delay84/1(d)
  pkt_reord_cnt1/1 -> Terminator31/1
  pkt_reord_err1/1 -> Terminator32/1
  Delay84/1(q) -> pkt_reord_cnt1/1
  Delay90/1(q) -> pkt_reord_err1/1
  pkt_reord1/6(mcnt_out) -> Delay52/1(d)
  pkt_reord1/1(sync_out) -> [x_sync1], miss_cnt1/4(sync), Delay49/1(d)
  pkt_reord1/2(data_out) -> Delay50/1(d)
  pkt_reord1/3(valid_out) -> Delay51/1(d), miss_cnt1/2(valid)
  pkt_reord1/5(rcvd) -> miss_cnt1/3(rcvd)
  xeng_tvg1/1(sync_out) -> Delay85/1(d)
  xeng_tvg1/2(data_out) -> Delay92/1(d)
  xeng_tvg1/3(valid_out) -> Delay94/1(d)
  xeng1/1(sync_out) -> vacc_tvg1/2(sync_in)
  xeng1/2(acc) -> 16b_32b1/1(In1)
  xeng1/3(valid) -> vacc_tvg1/4(valid_in)
  [xeng_tvg_sel] -> xeng_tvg1/1(tvg_sel)
  [vacc_tvg_sel] -> vacc_tvg1/1(tvg_sel)
  Delay30/1(q) -> Delay95/1(d)
  Delay49/1(q) -> xeng_tvg1/2(sync)
  Delay50/1(q) -> xeng_tvg1/3(data_in)
  Delay51/1(q) -> xeng_tvg1/4(valid_in)
  Delay52/1(q) -> Delay30/1(d)
  16b_32b1/1(Out1) -> vacc_tvg1/3(data_in)
  last_missing_ant1/1 -> Terminator28/1
  Delay28/1(q) -> last_missing_ant1/1
  miss_cnt1/3(last_missed_ant) -> Delay28/1(d)
  vacc_cnt1/1 -> Terminator20/1
  vacc_monitor1/2(spec_cnt) -> vacc_cnt1/1
  vacc_err_cnt1/1 -> Terminator8/1
  vacc_monitor1/1(error_cnt) -> vacc_err_cnt1/1
  [cnt_rst] -> vacc_monitor1/1(cnt_rst)
  vacc_mcnt_h1/1 -> Terminator29/1
  [vacc_mcnt1] -> Slice11/1
  Slice11/1 -> vacc_mcnt_h1/1
  vacc_mcnt_l1/1 -> Terminator34/1
  [vacc_mcnt1] -> Slice12/1
  Slice12/1 -> vacc_mcnt_l1/1
  demux_gbe0/1(valid) -> pkt_reord0/2(valid_in)
  demux_gbe1/1(valid) -> pkt_reord1/2(valid_in)
  Subsystem3/1(data_out) -> snap_gbe_tx0/1(data_in)
  Subsystem3/2(oob_out) -> snap_gbe_tx0/2(oob_in)
  Subsystem3/3(trig_out) -> snap_gbe_tx0/3(trig)
  Subsystem3/4(we_out) -> snap_gbe_tx0/4(we)
  [gbe_up] -> Subsystem3/5(link_up)
  [gbe_tx] -> Subsystem3/6(led_tx)
  [gbe_txd] -> Subsystem3/1(data_in)
  [gbe_txvld] -> Subsystem3/2(valid_in)
  [gbe_txip] -> Subsystem3/3(ip_in)
  [gbe_txeof] -> Subsystem3/4(eof_in)
  [gbe_rxvld] -> Delay25/1(d)
  [gbe_rxeof] -> Delay18/1(d)
  Delay25/1(q) -> Subsystem/2(valid)
  Delay18/1(q) -> Subsystem/3(eof)
  [gbe_rx_bad] -> Delay6/1(d)
  [gbe_rx_over] -> Delay7/1(d)
  [gbe_rxip] -> Delay53/1(d)
  [gbe_rxvld] -> Delay59/1(d)
  [gbe_rxdata] -> Delay70/1(d)
  [gbe_rxeof] -> Delay29/1(d)
  Delay70/1(q) -> Subsystem4/3(data_in)
  Delay59/1(q) -> Subsystem4/4(valid_in)
  Delay53/1(q) -> Subsystem4/5(ip_in)
  Delay29/1(q) -> Subsystem4/6(eof_in)
  [gbe_rxack] -> Subsystem4/9(ack_in)
  Delay80/1(q) -> Subsystem5/2(we)
  Delay81/1(q) -> Subsystem5/3(eof)
  Delay82/1(q) -> Subsystem5/4(flag)
  xaui_pack0/8(hdr) -> Terminator37/1
  xaui_pack0/9(hdr_valid) -> Terminator36/1
  Delay57/1(q) -> [xaui_valid]
  Delay55/1(q) -> [xaui_oob]
  Delay54/1(q) -> [xaui_data]
  Delay77/1(q) -> [xaui_link_dn]
  [xaui_data] -> Subsystem11/2(data)
  [xaui_oob] -> Subsystem11/3(oob)
  [xaui_valid] -> Subsystem11/4(valid)
  [xaui_data] -> Delay1/1(d)
  [xaui_oob] -> Delay3/1(d)
  [xaui_valid] -> Delay4/1(d)
  [xaui_link_dn] -> Delay5/1(d)
  Delay9/1(q) -> [xaui_pkt_data]
  Delay23/1(q) -> [xaui_pkt_valid]
  Delay10/1(q) -> [xaui_pkt_ip]
  Delay11/1(q) -> [xaui_pkt_eof]
  [xaui_pkt_valid] -> Logical1/1
  [gbe_base_ip] -> Delay46/1(d)
  Logical3/1 -> [gbe_rst_int]
  [gbe_rst] -> Logical3/2
  Constant20/1 -> Logical3/1
  [gbe_txd] -> Delay14/1(d)
  [gbe_txvld] -> Delay15/1(d)
  [gbe_txip] -> Delay16/1(d)
  [gbe_txeof] -> Delay44/1(d)
  [gbe_tx_discard] -> Delay45/1(d)
  Logical1/1 -> [gbe_txvld]
  [xaui_pkt_data] -> [gbe_txd]
  [xaui_pkt_ip] -> [gbe_txip]
  [xaui_pkt_eof] -> [gbe_txeof]
  [xaui_data] -> Subsystem9/1(data_in)
  [xaui_oob] -> Subsystem9/2(oob_in)
  [xaui_valid] -> Subsystem9/3(we_in)
  [xaui_link_dn] -> Subsystem9/4(link_down)
  [xaui_pkt_err] -> [gbe_tx_discard]
  [gbe_tx_discard] -> gbe_err/2(discard)
  [gbe_txeof] -> gbe_err/3(eof)
  [gbe_txvld] -> gbe_err/4(valid)
  Delay6/1(q) -> Subsystem/4(rx_bad_frame)
  Delay7/1(q) -> Subsystem/5(rx_overflow)
  [gbe_rx_bad] -> Delay8/1(d)
  [gbe_rx_over] -> Delay12/1(d)
  Delay14/1(q) -> loopback_fifo0/1(din)
  Delay15/1(q) -> loopback_fifo0/2(we)
  Delay16/1(q) -> loopback_fifo0/3(dest_ip)
  Delay44/1(q) -> loopback_fifo0/4(eof)
  Delay45/1(q) -> loopback_fifo0/5(discard)
  Delay46/1(q) -> loopback_fifo0/6(my_ip)
  Delay47/1 -> loopback_fifo0/7(re)
  Delay48/1 -> rx_pkt_fifo0/5(re)
  [gbe_tx_full] -> Subsystem3/7(tx_full)
  [gbe_tx_over] -> Subsystem3/8(tx_over)
  Delay32/1(q) -> pulse_ext3/1(in)
  Delay56/1(q) -> xeng0/1(sync_in)
  Delay73/1(q) -> xeng0/2(ant)
  Delay74/1(q) -> xeng0/3(window_valid)
  Delay83/1(q) -> xeng0/4(mcnt_in)
  Delay85/1(q) -> xeng1/1(sync_in)
  Delay92/1(q) -> xeng1/2(ant)
  Delay94/1(q) -> xeng1/3(window_valid)
  Delay95/1(q) -> xeng1/4(mcnt_in)
  [gbe_rst_int] -> gbe0/1
  [gbe_txd] -> gbe0/2
  [gbe_txvld] -> gbe0/3
  [gbe_txip] -> gbe0/4
  [gbe_txport] -> gbe0/5
  [gbe_txeof] -> gbe0/6
  [gbe_rxack] -> gbe0/7
  Constant2/1 -> [gbe_rxack]
  [gbe_rxack] -> gbe0/8
  gbe0/1 -> [gbe_up]
  gbe0/2 -> [gbe_rx]
  gbe0/3 -> [gbe_tx]
  gbe0/4 -> [gbe_tx_full]
  gbe0/5 -> [gbe_tx_over]
  gbe0/12 -> [gbe_rx_over]
  gbe0/11 -> [gbe_rx_bad]
  gbe0/6 -> [gbe_rxdata]
  gbe0/7 -> [gbe_rxvld]
  gbe0/8 -> [gbe_rxip]
  gbe0/9 -> [gbe_rxport]
  gbe0/10 -> [gbe_rxeof]
  Constant12/1 -> vacc_time_msw/1
  Constant6/1 -> vacc_time_lsw/1
  vacc_time_msw/1 -> Slice4/1, Slice13/1
  Slice13/1 -> Concat12/1
  vacc_time_lsw/1 -> Concat12/2
  Slice4/1 -> [vacc_ld_arm]
  Concat12/1 -> [vacc_ld_time]
  [vacc_ld_arm] -> vacc0/2(arm)
  [vacc_ld_time] -> vacc0/3(load_time)
  [vacc_rst] -> vacc0/1(rst)
  vacc0/9(ld_status) -> vacc_ld_status0/1
  vacc_ld_status0/1 -> Terminator12/1
  vacc_tvg1/1(sync_out) -> vacc1/5(sync_in)
  vacc_tvg1/2(data_out) -> vacc1/6(data_in)
  vacc_tvg1/3(valid_out) -> vacc1/7(we)
  [acc_len] -> vacc1/4(acc_len)
  vacc1/2(data_out) -> snap_vacc1/1(din)
  vacc1/4(valid_out) -> snap_vacc1/2(we)
  xeng1/4(mcnt_out) -> delay1/1
  snap_vacc1/1(fetch) -> vacc1/9(re)
  vacc1/8(vacc_mcnt) -> [vacc_mcnt1]
  [vacc_ld_arm] -> vacc1/2(arm)
  [vacc_ld_time] -> vacc1/3(load_time)
  [vacc_rst] -> vacc1/1(rst)
  vacc1/9(ld_status) -> vacc_ld_status1/1
  vacc_ld_status1/1 -> Terminator23/1
  delay5/1 -> vacc0/8(mcnt_in)
  delay1/1 -> vacc1/8(mcnt_in)
  vacc1/1(new_acc) -> [new_acc1]
  [new_acc1] -> vacc_monitor1/3(new_acc)
  vacc1/5(error_out) -> [vacc_err1]
  [vacc_err1] -> vacc_monitor1/2(error)
  vacc0/5(error_out) -> [vacc_err0]
  [new_acc0] -> vacc_monitor/2(new_acc)
  [vacc_err0] -> vacc_monitor/3(error)
